simulink model "SdbRxSigProc"  (R2022b)
settings: Solver=FixedStepDiscrete  StartTime=0.0  StopTime=10.0  FixedStep=0.05  RelTol=1e-3
(part 2 of 4)

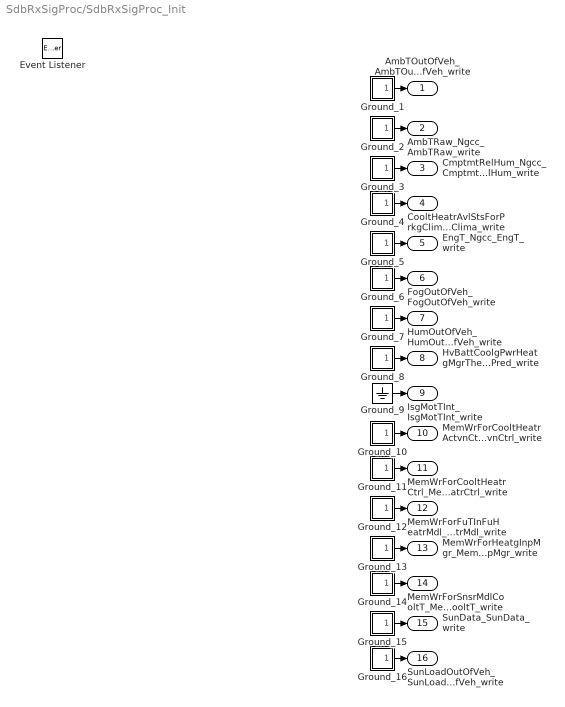
== system SdbRxSigProc/SdbRxSigProc_Init ==
  Event Listener : EventListener  EventName=reset
  Ground_1 : Subsystem
  Ground_10 : Subsystem
  Ground_11 : Subsystem
  Ground_12 : Subsystem
  Ground_13 : Subsystem
  Ground_14 : Subsystem
  Ground_15 : Subsystem
  Ground_16 : Subsystem
  Ground_2 : Subsystem
  Ground_3 : Subsystem
  Ground_4 : Subsystem
  Ground_5 : Subsystem
  Ground_6 : Subsystem
  Ground_7 : Subsystem
  Ground_8 : Subsystem
  Ground_9 : Ground
  AmbTOutOfVeh_AmbTOutOfVeh_write : Outport
  AmbTRaw_Ngcc_AmbTRaw_write : Outport  Port=2
  CmptmtRelHum_Ngcc_CmptmtRelHum_write : Outport  Port=3
  CooltHeatrAvlStsForPrkgClima_CooltHeatrAvlStsForPrkgClima_write : Outport  Port=4
  EngT_Ngcc_EngT_write : Outport  Port=5
  FogOutOfVeh_FogOutOfVeh_write : Outport  Port=6
  HumOutOfVeh_HumOutOfVeh_write : Outport  Port=7
  HvBattCoolgPwrHeatgMgrThermPred_HvBattCoolgPwrHeatgMgrThermPred_write : Outport  Port=8
  IsgMotTInt_IsgMotTInt_write : Outport  Port=9
  MemWrForCooltHeatrActvnCtrl_MemWrForCooltHeatrActvnCtrl_write : Outport  Port=10
  MemWrForCooltHeatrCtrl_MemWrForCooltHeatrCtrl_write : Outport  Port=11
  MemWrForFuTInFuHeatrMdl_MemWrForFuTInFuHeatrMdl_write : Outport  Port=12
  MemWrForHeatgInpMgr_MemWrForHeatgInpMgr_write : Outport  Port=13
  MemWrForSnsrMdlCooltT_MemWrForSnsrMdlCooltT_write : Outport  Port=14
  SunData_SunData_write : Outport  Port=15
  SunLoadOutOfVeh_SunLoadOutOfVeh_write : Outport  Port=16
  wires:
  Ground_1/1(Out1) -> AmbTOutOfVeh_AmbTOutOfVeh_write/1
  Ground_2/1(Out1) -> AmbTRaw_Ngcc_AmbTRaw_write/1
  Ground_3/1(Out1) -> CmptmtRelHum_Ngcc_CmptmtRelHum_write/1
  Ground_4/1(Out1) -> CooltHeatrAvlStsForPrkgClima_CooltHeatrAvlStsForPrkgClima_write/1
  Ground_5/1(Out1) -> EngT_Ngcc_EngT_write/1
  Ground_6/1(Out1) -> FogOutOfVeh_FogOutOfVeh_write/1
  Ground_7/1(Out1) -> HumOutOfVeh_HumOutOfVeh_write/1
  Ground_8/1(Out1) -> HvBattCoolgPwrHeatgMgrThermPred_HvBattCoolgPwrHeatgMgrThermPred_write/1
  Ground_9/1 -> IsgMotTInt_IsgMotTInt_write/1
  Ground_10/1(Out1) -> MemWrForCooltHeatrActvnCtrl_MemWrForCooltHeatrActvnCtrl_write/1
  Ground_11/1(Out1) -> MemWrForCooltHeatrCtrl_MemWrForCooltHeatrCtrl_write/1
  Ground_12/1(Out1) -> MemWrForFuTInFuHeatrMdl_MemWrForFuTInFuHeatrMdl_write/1
  Ground_13/1(Out1) -> MemWrForHeatgInpMgr_MemWrForHeatgInpMgr_write/1
  Ground_14/1(Out1) -> MemWrForSnsrMdlCooltT_MemWrForSnsrMdlCooltT_write/1
  Ground_15/1(Out1) -> SunData_SunData_write/1
  Ground_16/1(Out1) -> SunLoadOutOfVeh_SunLoadOutOfVeh_write/1
